simulink model "MCVT_Integration"  (R2020a)
settings: Solver=ode8  StartTime=0.0  StopTime=T_end  FixedStep=dt  RelTol=1e-3  EnableMultiTasking=on
values from neighbouring files (symbols this model uses):
  assumed_power_voltage = 1.25e2    (= 125)    [MCVT_Input_File.m]    (also in MCVT_Run_QuickCheck.m)
  battery_power_toggle = 1    [MCVT_Input_File.m]    (also in MCVT_Run_QuickCheck.m)
  battery_trickle_charge_param = 0.1    [MCVT_Input_File.m]    (also in MCVT_Run_QuickCheck.m)
  battery_trickle_leakage_param = 0.01    [MCVT_Input_File.m]    (also in MCVT_Run_QuickCheck.m)
  damage_case_id = 3    [MCVT_Input_File.m]    (also in MCVT_Run_QuickCheck.m = 1)
  dt = 1/fs    (= 0.001)    [MCVT_Run_QuickCheck.m]    (also in MCVT_Sim_Set.m)
  dt_2 = 1/fs_2    (= 0.001)    [MCVT_Run_QuickCheck.m]    (also in MCVT_Sim_Set.m)
  dt_3 = 1/fs_3    (= 0.001)    [MCVT_Run_QuickCheck.m]    (also in MCVT_Sim_Set.m)
  dt_5 = 1/fs_5    (= 0.005)    [MCVT_Run_QuickCheck.m]    (also in MCVT_Sim_Set.m)
  dt_8 = 1/fs_8    (= 0.05)    [MCVT_Run_QuickCheck.m]    (also in MCVT_Sim_Set.m)
  dt_9 = 1/fs_9    (= 0.001)    [MCVT_Run_QuickCheck.m]    (also in MCVT_Sim_Set.m)
  dust_met_duration = 3    [MCVT_Input_File.m]    (also in MCVT_Run_QuickCheck.m = 2)
  ECLSS_indoor_set_temperature = 298.15    [MCVT_Input_File.m]    (also in MCVT_Run_QuickCheck.m)
  energy_store_max_o = 150    [MCVT_Input_File.m]    (also in MCVT_Run_QuickCheck.m)
  energy_store_o = 120    [MCVT_Input_File.m]    (also in MCVT_Run_QuickCheck.m)
  fs = 1000    [MCVT_Run_QuickCheck.m]    (also in MCVT_Sim_Set.m)
  gain_charge_param = 1    [MCVT_Input_File.m]    (also in MCVT_Run_QuickCheck.m)
  ground_temperature = 373    [MCVT_Habitat_Design_Input_File.m]    (also in MCVT_Run_QuickCheck.m)
  I_max_charge = 800    [MCVT_Input_File.m]    (also in MCVT_Run_QuickCheck.m)
  I_max_discharge = 800    [MCVT_Input_File.m]    (also in MCVT_Run_QuickCheck.m)
  initial_pressure = 1e5    (= 100000)    [MCVT_Input_File.m]    (also in MCVT_Run_QuickCheck.m)
  initial_temperature = 295    [MCVT_Input_File.m]    (also in MCVT_Run_QuickCheck.m)
  interior_leak_rate = 0.00415    [MCVT_Input_File.m]    (also in MCVT_Run_QuickCheck.m)
  launch_dust_accum_start_time = 10    [MCVT_Input_File.m]    (also in MCVT_Run_QuickCheck.m = 2)
  launch_dust_accum_stop_time = 12    [MCVT_Input_File.m]    (also in MCVT_Run_QuickCheck.m = 3)
  meteorite_impact_time = 2    [MCVT_Input_File.m]    (also in MCVT_Run_QuickCheck.m = 1)
  nuclear_power_toggle = 1    [MCVT_Input_File.m]    (also in MCVT_Run_QuickCheck.m)
  print_interval = 30    [MCVT_Input_File.m]
  SOC_lower_thres = 0.5    [MCVT_Input_File.m]    (also in MCVT_Run_QuickCheck.m)
  SOC_upper_thres = 0.9    [MCVT_Input_File.m]    (also in MCVT_Run_QuickCheck.m)
  solar_power_toggle = 1    [MCVT_Input_File.m]    (also in MCVT_Run_QuickCheck.m)
  space_temperature = 4    [MCVT_Habitat_Design_Input_File.m]    (also in MCVT_Run_QuickCheck.m)
  Start_angle = 90    [MCVT_Input_File.m]    (also in MCVT_Run_QuickCheck.m)
undefined symbols (not defined in the file or in its folder): A_flow_restrictor, ACTIVITY_LOOKUP_TABLE, air, Aref, Bmap, Bmap2, Bmap3, comms, DiscIntNeverNeededParam, DmgSPL, dust_parameters, ECLSS_dust_damage_repair_time, ECLSS_heater_capacity, ECLSS_IPS_restrictor, ECLSS_IPS_setpointPressure, ECLSS_paint_damage_repair_time, ECLSS_radiatorLoop_discreteNumber, ECLSS_Vst, emptyActivity, expected_heat_add, FI, habitat, HIEM2SMM, HIEM2STM, HIEMDamageElements, impact_numbering, initial_SPLT_damage_index, input_air, input_Cond, input_CP, input_discretization, input_EV, input_Evap, input_radiatorLoop, K_ECLSS_dust_single, K_ECLSS_paint_single, K_Structure_damage_single, Kref, kW_to_W, meteoroidDustDep, mFRF, ndelays, nominalDustDep, noverlap, nuclear_toggle_gain, output_limits, panel_paint0, Ps0, reference_temperature, RepairTime_ECLSS_factor, Sensor_Location_SMM, SMM_T0, SMM_T1, SMM_T2, SMM_T3, SMM_T4, SMM_T4_0, SMM_T4_1, SMM_T4_2, solar_FDD_K ... (+73)
libraries: neural x350, simulink x39, xpcutilitieslib x7, xpcrtudplib x7, dspstat3 x1
(part 20 of 43)

== system MCVT_Integration/Sys2 - Structural System/Sys2_Structural_System/Fault Detection and Diagnosis (FDD) 2.3/Pattern Recognition Neural Network/Pattern Recognition Neural Network with 10% Noise/Layer 2/Delays 1 ==
  "p{2}" : Inport  PortDimensions=20
      mask Display: e = 0.06;c1 = [120 230 180]/255;c2 = ([120 230 180]+0)/(256+128);c3 = ([120 230 180]+128)/(256+128);patch([0 1 1 0],[0 0 1 1],c1);patch([0 1 1 1-e 1-e 0+e],[0 0 1 1-e 0+e 0+e],c2);patch([0 0 1 1-e 0+e 0+e],[0 1 1 1-e 1-e 0+e],c3);plot([0 1 1 0 0],[0 0 1 1 0]);
  mux : Mux  Inputs=1
      mask Display: e = 0.06;c1 = [120 150 230]/255;c2 = ([120 150 230]+0)/383;c3 = ([120 150 230]+128)/383;patch([0 1 1 0],[0 0 1 1],c1);patch([0 1 1 1-e 1-e 0+e],[0 0 1 1-e 0+e 0+e],c2);patch([0 0 1 1-e 0+e 0+e],[0 1 1 1-e 1-e 0+e],c3);plot([0 1 1 0 0],[0 0 1 1 0]);disp('Mux')
  "pd{2,1}" : Outport
      mask Display: e = 0.06;c1 = [120 230 180]/255;c2 = ([120 230 180]+0)/(256+128);c3 = ([120 230 180]+128)/(256+128);patch([0 1 1 0],[0 0 1 1],c1);patch([0 1 1 1-e 1-e 0+e],[0 0 1 1-e 0+e 0+e],c2);patch([0 0 1 1-e 0+e 0+e],[0 1 1 1-e 1-e 0+e],c3);plot([0 1 1 0 0],[0 0 1 1 0]);
  wires:
  "p{2}"/1 -> mux/1
  mux/1 -> "pd{2,1}"/1

== system MCVT_Integration/Sys2 - Structural System/Sys2_Structural_System/Fault Detection and Diagnosis (FDD) 2.3/Pattern Recognition Neural Network/Pattern Recognition Neural Network with 10% Noise/Layer 2/LW{2,1} ==
  "ad{2,1}" : Inport  PortDimensions=20
      mask Display: e = 0.06;c1 = [120 230 180]/255;c2 = ([120 230 180]+0)/(256+128);c3 = ([120 230 180]+128)/(256+128);patch([0 1 1 0],[0 0 1 1],c1);patch([0 1 1 1-e 1-e 0+e],[0 0 1 1-e 0+e 0+e],c2);patch([0 0 1 1-e 0+e 0+e],[0 1 1 1-e 1-e 0+e],c3);plot([0 1 1 0 0],[0 0 1 1 0]);
  "IW{2,1}(1,:)'" : Constant  Value=[-0.574271628074736195657123971614055335521697998046875;0.50810117268876220375517505090101622045040130615234375;-0.9283778525542050186203368866699747741222381591796875;-0.032683118602653167539262568652702611871063709259033203125;-0.0145954644987950805934406872665931587107479572296142578125;0.443944394435375422336420569990877993404865264892578125;0.4214764366206338319642554779420606791973114013671875;-0.386523310467667136069991329350159503519535064697265625;-0.25850103440934268927975381302530877292156219482421875;-0.91087816091310769284206116935820318758487701416015625;-0.1963259746056559562621401937576592899858951568603515625;-0.1813018586134855503200213888703729026019573211669921875;-0.49254604106108257344232015384477563202381134033203125;-0.394272569002943440441555367215187288820743560791015625;-0.062085423077329747465480380697044893167912960052490234375;-0.041503725787217782272620070216362364590167999267578125;0.1806376114545178435122352311736904084682464599609375;0.164222888558606339159950948669575154781341552734375;-0.5222337211202112694508059576037339866161346435546875;-0.441330444361871021552445881752646528184413909912109375]
      mask Display: e = 0.06;c1 = [120 150 230]/255;c2 = ([120 150 230]+0)/383;c3 = ([120 150 230]+128)/383;patch([0 1 1 0],[0 0 1 1],c1);patch([0 1 1 1-e 1-e 0+e],[0 0 1 1-e 0+e 0+e],c2);patch([0 0 1 1-e 0+e 0+e],[0 1 1 1-e 1-e 0+e],c3);plot([0 1 1 0 0],[0 0 1 1 0]);disp('weights');
  "IW{2,1}(10,:)'" : Constant  Value=[0.56889257212312560607614386753994040191173553466796875;0.1885837971524775558140873954471317119896411895751953125;0.6485295796017667147026486418326385319232940673828125;-0.451249687405113453220195651738322339951992034912109375;-0.3971195859441050135529849285376258194446563720703125;0.427965433320891597279711504597798921167850494384765625;-0.656993381392033892751669554854743182659149169921875;-0.0936009421839196498194013429383630864322185516357421875;-0.234845048198842609732395203536725603044033050537109375;-0.84261949124741220007450692719430662691593170166015625;0.3092932033834203675581875359057448804378509521484375;-0.69446240960240535056158250881708227097988128662109375;0.133242084727271781208202128254924900829792022705078125;0.49326867993430412173694321609218604862689971923828125;-0.06733328074539456087332922606947249732911586761474609375;-0.278535206843569416168548968926188535988330841064453125;0.277173212586397099688184653132339008152484893798828125;0.168172076864115560557166872968082316219806671142578125;-0.338431949921502994715893919419613666832447052001953125;0.020035112474400061655099847257588407956063747406005859375]
      mask Display: e = 0.06;c1 = [120 150 230]/255;c2 = ([120 150 230]+0)/383;c3 = ([120 150 230]+128)/383;patch([0 1 1 0],[0 0 1 1],c1);patch([0 1 1 1-e 1-e 0+e],[0 0 1 1-e 0+e 0+e],c2);patch([0 0 1 1-e 0+e 0+e],[0 1 1 1-e 1-e 0+e],c3);plot([0 1 1 0 0],[0 0 1 1 0]);disp('weights');
  "IW{2,1}(11,:)'" : Constant  Value=[-0.61220927358305521348569300243980251252651214599609375;-0.35057890410002789938204159625456668436527252197265625;-0.523626035897452712930544294067658483982086181640625;0.436194545470680983267897090627229772508144378662109375;0.1213313887947793101584892383471014909446239471435546875;-0.0121676389988455875557082919158347067423164844512939453125;-0.0633751033433716803866531108724302612245082855224609375;-0.54877334524708165641726509420550428330898284912109375;0.1756665111292425029976271844134316779673099517822265625;0.52479855734203051387254390647285617887973785400390625;0.31253261939911680666881466095219366252422332763671875;-0.061689810511481708854564232069606077857315540313720703125;-0.36854617261526312343988820430240593850612640380859375;0.400723045351511986122972075463621877133846282958984375;-0.1532168475451168021539416486120899207890033721923828125;-0.64645781400316526532634497925755567848682403564453125;0.5697695890396656803744690478197298943996429443359375;0.1448942726284534188163632961732218973338603973388671875;-0.438188292108895705023741129480185918509960174560546875;-0.2074698900457048467149689940924872644245624542236328125]
      mask Display: e = 0.06;c1 = [120 150 230]/255;c2 = ([120 150 230]+0)/383;c3 = ([120 150 230]+128)/383;patch([0 1 1 0],[0 0 1 1],c1);patch([0 1 1 1-e 1-e 0+e],[0 0 1 1-e 0+e 0+e],c2);patch([0 0 1 1-e 0+e 0+e],[0 1 1 1-e 1-e 0+e],c3);plot([0 1 1 0 0],[0 0 1 1 0]);disp('weights');
  "IW{2,1}(12,:)'" : Constant  Value=[-0.05684625507318873116613389129270217381417751312255859375;0.7124306870197238961139873936190269887447357177734375;-0.0255329045034950428971765035157659440301358699798583984375;0.2385497059408359932231036282246350310742855072021484375;0.97337720939202998504669039903092198073863983154296875;0.3646568944777561771530827172682620584964752197265625;0.54797761780701914613445069335284642875194549560546875;0.37164823961946014119206438408582471311092376708984375;-0.08512149605261863649641185247674002312123775482177734375;-0.09309100636109647608495976101039559580385684967041015625;0.10445071447821853427573302042219438590109348297119140625;0.450251121221431793717471236959681846201419830322265625;-0.315036492181973659665317200051504187285900115966796875;0.2069138675097972335237273000529967248439788818359375;-0.07269256325197571977358990125139825977385044097900390625;-0.358315402073627942147737712730304338037967681884765625;0.00748683608178967417712268428431343636475503444671630859375;0.63657745039067881176464425152516923844814300537109375;-0.0188097270602836132147306358319838182069361209869384765625;-0.435424821932042849237376458404469303786754608154296875]
      mask Display: e = 0.06;c1 = [120 150 230]/255;c2 = ([120 150 230]+0)/383;c3 = ([120 150 230]+128)/383;patch([0 1 1 0],[0 0 1 1],c1);patch([0 1 1 1-e 1-e 0+e],[0 0 1 1-e 0+e 0+e],c2);patch([0 0 1 1-e 0+e 0+e],[0 1 1 1-e 1-e 0+e],c3);plot([0 1 1 0 0],[0 0 1 1 0]);disp('weights');
  "IW{2,1}(13,:)'" : Constant  Value=[-0.005992500139532979351597585804256596020422875881195068359375;-0.4560900715520570525285393159720115363597869873046875;-0.306276633508657025739552182130864821374416351318359375;0.5216871924691381945393686692113988101482391357421875;-0.316654318650212707186852867380366660654544830322265625;0.1121328110068039174418430548030300997197628021240234375;0.093640236792591424741516448193578980863094329833984375;0.17502083406653123898166768412920646369457244873046875;-0.60837609782212742626228418885148130357265472412109375;0.357162613418083640937084055622108280658721923828125;0.64597875254259007338220044402987696230411529541015625;-0.412303583049930477688604923969251103699207305908203125;-0.29760774376247056682842639929731376469135284423828125;-0.306518613632401681901029633081634528934955596923828125;0.26479518516167266373173561078147031366825103759765625;0.1475845467874192518120679551429930143058300018310546875;-0.273480753422654820905535189012880437076091766357421875;0.634244403850074522921431707800365984439849853515625;-0.2405905212879165533390590780982165597379207611083984375;0.042143875875917773121681619841183419339358806610107421875]
      mask Display: e = 0.06;c1 = [120 150 230]/255;c2 = ([120 150 230]+0)/383;c3 = ([120 150 230]+128)/383;patch([0 1 1 0],[0 0 1 1],c1);patch([0 1 1 1-e 1-e 0+e],[0 0 1 1-e 0+e 0+e],c2);patch([0 0 1 1-e 0+e 0+e],[0 1 1 1-e 1-e 0+e],c3);plot([0 1 1 0 0],[0 0 1 1 0]);disp('weights');
  "IW{2,1}(14,:)'" : Constant  Value=[0.83050427742647514595120128433336503803730010986328125;0.380791907424027631368090851537999697029590606689453125;0.64991803081990628943032106690225191414356231689453125;-0.08751040212893225478385517135393456555902957916259765625;-0.304332767679233062718680002944893203675746917724609375;-0.54612464997613496819894862710498273372650146484375;-0.35114844609709716660717049308004789054393768310546875;-0.348036230611717234406654597478336654603481292724609375;-0.53144460799473625112199215436703525483608245849609375;-0.0777327876198296296994527665447094477713108062744140625;-0.345073957804460318410377794862142764031887054443359375;-0.205600532452644924052975738959503360092639923095703125;-0.049181910233272840837770445432397536933422088623046875;0.250885063190241563990667827965808100998401641845703125;0.43391138223406156004813283288967795670032501220703125;-0.1461886322383406378033754435818991623818874359130859375;0.6178452278896970906174601623206399381160736083984375;0.266994784317448774491055019097984768450260162353515625;-0.49335961290386498578897089828387834131717681884765625;0.022843510039939569911116024059083429165184497833251953125]
      mask Display: e = 0.06;c1 = [120 150 230]/255;c2 = ([120 150 230]+0)/383;c3 = ([120 150 230]+128)/383;patch([0 1 1 0],[0 0 1 1],c1);patch([0 1 1 1-e 1-e 0+e],[0 0 1 1-e 0+e 0+e],c2);patch([0 0 1 1-e 0+e 0+e],[0 1 1 1-e 1-e 0+e],c3);plot([0 1 1 0 0],[0 0 1 1 0]);disp('weights');
  "IW{2,1}(15,:)'" : Constant  Value=[0.394338359224950363302042433133465237915515899658203125;-0.324812785108549439971881156452582217752933502197265625;0.0334474272752659229634986104429117403924465179443359375;-0.113914753942840007994163897819817066192626953125;0.5702661865720821676717378068133257329463958740234375;-0.56902393479980106771876080529182218015193939208984375;-1.080364855197034845701864469447173178195953369140625;-0.43915404493278786812737735090195201337337493896484375;0.02888946354385936443609494972406537272036075592041015625;0.360330786452452256352074755341163836419582366943359375;-0.472775036000186776874443239648826420307159423828125;0.5191143553324497617040833574719727039337158203125;0.05346616409203776287828446811545290984213352203369140625;-0.108658401740322874129418551092385314404964447021484375;0.67532661092200696462128917119116522371768951416015625;-0.5013587075398808767801028807298280298709869384765625;0.393844819705647442020790549577213823795318603515625;0.412756827570472217825425786941195838153362274169921875;-0.55847529512976767929188781636185012757778167724609375;-0.205862070370640803940176510877790860831737518310546875]
      mask Display: e = 0.06;c1 = [120 150 230]/255;c2 = ([120 150 230]+0)/383;c3 = ([120 150 230]+128)/383;patch([0 1 1 0],[0 0 1 1],c1);patch([0 1 1 1-e 1-e 0+e],[0 0 1 1-e 0+e 0+e],c2);patch([0 0 1 1-e 0+e 0+e],[0 1 1 1-e 1-e 0+e],c3);plot([0 1 1 0 0],[0 0 1 1 0]);disp('weights');
  "IW{2,1}(16,:)'" : Constant  Value=[0.27635654239410623045358761373790912330150604248046875;-0.04429311840556114654443575773257180117070674896240234375;0.5431988410510484310833589916001074016094207763671875;-0.57140306934570206376378109780489467084407806396484375;0.28768730719099533388316558557562530040740966796875;0.5939455878345356598657645008643157780170440673828125;0.07670371326740492434748119876530836336314678192138671875;0.592025013385914622432437681709416210651397705078125;-0.12346456437285503737744107866092235781252384185791015625;0.40383872281908173018649677032954059541225433349609375;0.1638374993707522320196545706494362093508243560791015625;-0.233077013410165623241709909052588045597076416015625;-0.382987569183739273714905948509112931787967681884765625;0.237088361565319261092810165791888721287250518798828125;-0.032614993468910190299592244400628260336816310882568359375;0.18972721693147354127262360634631477296352386474609375;0.477835629992515276143194569158367812633514404296875;-0.444967139434641223427746581364772282540798187255859375;0.36956516035198128111716187049751169979572296142578125;0.260159387876649272897111586644314229488372802734375]
      mask Display: e = 0.06;c1 = [120 150 230]/255;c2 = ([120 150 230]+0)/383;c3 = ([120 150 230]+128)/383;patch([0 1 1 0],[0 0 1 1],c1);patch([0 1 1 1-e 1-e 0+e],[0 0 1 1-e 0+e 0+e],c2);patch([0 0 1 1-e 0+e 0+e],[0 1 1 1-e 1-e 0+e],c3);plot([0 1 1 0 0],[0 0 1 1 0]);disp('weights');
  "IW{2,1}(17,:)'" : Constant  Value=[-0.10300827207069433721908779943987610749900341033935546875;-0.062365882473237134464572051228969939984381198883056640625;-0.0506656936086894160720106583539745770394802093505859375;0.400652635851392491250777538880356587469577789306640625;-0.64362923376520597695815695260534994304180145263671875;-0.10550968910703635572634340178410639055073261260986328125;-0.436641866892334473693182417264324612915515899658203125;0.2877789503365608680951481801457703113555908203125;-0.0856422481667453439069248588566551916301250457763671875;-0.0352973610691887651480413978788419626653194427490234375;0.251271296193900373072693810172495432198047637939453125;0.158396296935443603448590010884799994528293609619140625;-0.02336399796777975657047221602624631486833095550537109375;0.2405152682774551464728318705965648405253887176513671875;-0.422851332433710036529106446323567070066928863525390625;0.0545271257407160536700985176139511168003082275390625;0.63574596562661944698646721008117310702800750732421875;0.71382341994933418138913339134887792170047760009765625;-0.5918051425522603548046163268736563622951507568359375;0.18430848892072082545467992531484924256801605224609375]
      mask Display: e = 0.06;c1 = [120 150 230]/255;c2 = ([120 150 230]+0)/383;c3 = ([120 150 230]+128)/383;patch([0 1 1 0],[0 0 1 1],c1);patch([0 1 1 1-e 1-e 0+e],[0 0 1 1-e 0+e 0+e],c2);patch([0 0 1 1-e 0+e 0+e],[0 1 1 1-e 1-e 0+e],c3);plot([0 1 1 0 0],[0 0 1 1 0]);disp('weights');
  "IW{2,1}(18,:)'" : Constant  Value=[-0.25446115074283692791823341394774615764617919921875;-0.476422817454355607136307071414194069802761077880859375;-0.48243039010070898342519285506568849086761474609375;0.12240083511392434167941445366523112170398235321044921875;0.370582478256486547163461864329292438924312591552734375;-0.565112313381039399473593221046030521392822265625;0.1811578060472032458694258139075827784836292266845703125;-0.385885379099205449993092997829080559313297271728515625;0.1234837116886016994410368852186365984380245208740234375;0.271571689696240070599486671198974363505840301513671875;0.26568034152349895293809822760522365570068359375;-0.36135745586302314702464855145080946385860443115234375;-0.2479618589940788542236305147525854408740997314453125;-0.465725675460194776800193494636914692819118499755859375;-0.029786737886606184932958285571658052504062652587890625;0.10761968787180224704780329147979500703513622283935546875;-0.39997554837126070292896429236861877143383026123046875;0.47018260234583897361204662956879474222660064697265625;0.474125103036238948472913534715189598500728607177734375;0.5711459194855550425273804648895747959613800048828125]
      mask Display: e = 0.06;c1 = [120 150 230]/255;c2 = ([120 150 230]+0)/383;c3 = ([120 150 230]+128)/383;patch([0 1 1 0],[0 0 1 1],c1);patch([0 1 1 1-e 1-e 0+e],[0 0 1 1-e 0+e 0+e],c2);patch([0 0 1 1-e 0+e 0+e],[0 1 1 1-e 1-e 0+e],c3);plot([0 1 1 0 0],[0 0 1 1 0]);disp('weights');
  "IW{2,1}(19,:)'" : Constant  Value=[-0.63386779810769622311994453411898575723171234130859375;-0.50314690781331872226900259192916564643383026123046875;0.268331211259087709652959574668784625828266143798828125;-0.1955754337239898887901290436275303363800048828125;-0.35669681164923139338185364977107383310794830322265625;0.502236421766609542061132742674089968204498291015625;-0.11159206649818120826722633864847011864185333251953125;0.436217158032130292166783647189731709659099578857421875;-0.27584823963679705460805280381464399397373199462890625;0.418362204883860833160014180975849740207195281982421875;-0.1899008077758812529367560273385606706142425537109375;0.57483413850714948356568356757634319365024566650390625;-0.446857134984141424194348246601293794810771942138671875;0.396945475581674422915767763697658665478229522705078125;-1.0613420562751685327640416289796121418476104736328125;-0.285221282032167178233095228279125876724720001220703125;0.45693712919073814493486906940233893692493438720703125;0.07744397689539132823011158279769006185233592987060546875;0.354164362297970491955112493087654002010822296142578125;-0.0162778031372035510149220982611950603313744068145751953125]
      mask Display: e = 0.06;c1 = [120 150 230]/255;c2 = ([120 150 230]+0)/383;c3 = ([120 150 230]+128)/383;patch([0 1 1 0],[0 0 1 1],c1);patch([0 1 1 1-e 1-e 0+e],[0 0 1 1-e 0+e 0+e],c2);patch([0 0 1 1-e 0+e 0+e],[0 1 1 1-e 1-e 0+e],c3);plot([0 1 1 0 0],[0 0 1 1 0]);disp('weights');
  "IW{2,1}(2,:)'" : Constant  Value=[0.170738862388308543227566360656055621802806854248046875;-0.268815089772659943090360457063070498406887054443359375;-0.38720939538457821438299788496806286275386810302734375;0.01483966791631497784964555108899730839766561985015869140625;-0.486697009966023108606947289445088244974613189697265625;0.309172138529930418116009605000726878643035888671875;0.06373514748131080265292069952920428477227687835693359375;-0.60590099369459993194908520308672450482845306396484375;0.35640677444520363348345881604473106563091278076171875;-0.5909803027849054313236365487682633101940155029296875;-0.1285903836946735712132294793264009058475494384765625;0.4466134666669081543233232878264971077442169189453125;-0.1984531065465708066231087514097453095018863677978515625;0.325192889511320359918755684702773578464984893798828125;0.47371923459628739738747071896796114742755889892578125;0.593039767991454969120468376786448061466217041015625;0.345064373904851395824522342081763781607151031494140625;0.1563268051332172559053645954918465577065944671630859375;0.2085629962423511674085574441050994209945201873779296875;0.2477676431329221828914199932114570401608943939208984375]
      mask Display: e = 0.06;c1 = [120 150 230]/255;c2 = ([120 150 230]+0)/383;c3 = ([120 150 230]+128)/383;patch([0 1 1 0],[0 0 1 1],c1);patch([0 1 1 1-e 1-e 0+e],[0 0 1 1-e 0+e 0+e],c2);patch([0 0 1 1-e 0+e 0+e],[0 1 1 1-e 1-e 0+e],c3);plot([0 1 1 0 0],[0 0 1 1 0]);disp('weights');
  "IW{2,1}(20,:)'" : Constant  Value=[0.034625411356367034876324595416008378379046916961669921875;0.1874616562450983348053767940655234269797801971435546875;0.04914463536819181632697706163526163436472415924072265625;-0.59175777311133870295378756054560653865337371826171875;-0.90960536825278170969255597810843028128147125244140625;0.32990973138561152655512387354974634945392608642578125;0.06897862063135924703960455417472985573112964630126953125;0.3352281455569865453725242332438938319683074951171875;0.56131365538922206592786778855952434241771697998046875;0.5368981405184618527215434369281865656375885009765625;-0.332503821804824628571850553271360695362091064453125;0.277925405078486231769119285672786645591259002685546875;-0.3078968918083386796524791861884295940399169921875;0.423244800153884437321494260686449706554412841796875;-0.492707237515911000702573119269800372421741485595703125;-0.124415208625734130531981236345018260180950164794921875;0.401670717019710299400259145841118879616260528564453125;0.254209817383537262092119135559187270700931549072265625;-0.335112590406773491746861282081226818263530731201171875;0.69289987289486221211376459905295632779598236083984375]
      mask Display: e = 0.06;c1 = [120 150 230]/255;c2 = ([120 150 230]+0)/383;c3 = ([120 150 230]+128)/383;patch([0 1 1 0],[0 0 1 1],c1);patch([0 1 1 1-e 1-e 0+e],[0 0 1 1-e 0+e 0+e],c2);patch([0 0 1 1-e 0+e 0+e],[0 1 1 1-e 1-e 0+e],c3);plot([0 1 1 0 0],[0 0 1 1 0]);disp('weights');
  "IW{2,1}(3,:)'" : Constant  Value=[-0.68384728468662192568672253401018679141998291015625;-0.18667975716109364014982929802499711513519287109375;-0.5796974119018820648108203386073000729084014892578125;0.444457322623959816265681865843362174928188323974609375;0.3358991937804882699225572650902904570102691650390625;0.62509683106080216052902187584550119936466217041015625;-0.2121453725660192024538019950341549701988697052001953125;0.1528299410147090087175314465639530681073665618896484375;-0.26522546290072701591356008066213689744472503662109375;-0.833329512393990778917896022903732955455780029296875;0.0245823899162304800036782381766897742636501789093017578125;0.3242450158499738943618240227806381881237030029296875;0.207550979496584797612257489163312129676342010498046875;-0.5781973676120577554371493533835746347904205322265625;0.84734773074372016221644798861234448850154876708984375;0.08584960360003891788327479162035160697996616363525390625;-0.5281438899797261665725045531871728599071502685546875;-0.377490389258728520349706059278105385601520538330078125;-0.255276769465087205457365371330524794757366180419921875;0.397482914908264428621720298906438983976840972900390625]
      mask Display: e = 0.06;c1 = [120 150 230]/255;c2 = ([120 150 230]+0)/383;c3 = ([120 150 230]+128)/383;patch([0 1 1 0],[0 0 1 1],c1);patch([0 1 1 1-e 1-e 0+e],[0 0 1 1-e 0+e 0+e],c2);patch([0 0 1 1-e 0+e 0+e],[0 1 1 1-e 1-e 0+e],c3);plot([0 1 1 0 0],[0 0 1 1 0]);disp('weights');
  "IW{2,1}(4,:)'" : Constant  Value=[-0.469126351790188966806027792699751444160938262939453125;0.65369714251824551087821646433440037071704864501953125;-0.62799203253491719944889837279333733022212982177734375;-0.2351882475270932193467388060525991022586822509765625;-0.11164975269919773237514704078421345911920070648193359375;-0.493471669872333873474445908868801780045032501220703125;0.9180235431853904781718256344902329146862030029296875;0.07102998928975334802515817500534467399120330810546875;0.316111646796484568877616538884467445313930511474609375;0.47279397677343915074033020573551766574382781982421875;0.39848637281463583637020064998068846762180328369140625;-0.85560991819153253690188876134925521910190582275390625;-0.415615780819191649175792235837434418499469757080078125;0.32152173865090272197875265192124061286449432373046875;0.58442540995306024864675009666825644671916961669921875;-0.1628689347883164606489714287818060256540775299072265625;-0.2123151870600946955480736733079538680613040924072265625;0.36373178934589844857327989302575588226318359375;-0.096673513630073759639316222092020325362682342529296875;-0.423399450849545611053059701589518226683139801025390625]
      mask Display: e = 0.06;c1 = [120 150 230]/255;c2 = ([120 150 230]+0)/383;c3 = ([120 150 230]+128)/383;patch([0 1 1 0],[0 0 1 1],c1);patch([0 1 1 1-e 1-e 0+e],[0 0 1 1-e 0+e 0+e],c2);patch([0 0 1 1-e 0+e 0+e],[0 1 1 1-e 1-e 0+e],c3);plot([0 1 1 0 0],[0 0 1 1 0]);disp('weights');
  "IW{2,1}(5,:)'" : Constant  Value=[0.467549098114494021682929769667680375277996063232421875;0.827698304288310371390480213449336588382720947265625;0.702652589865448984340901006362400949001312255859375;-0.51130807898153129986695830666576512157917022705078125;0.6848509081607254689316732765291817486286163330078125;0.3376259025690153681154015430365689098834991455078125;0.519716199692204394722239157999865710735321044921875;0.28040092188565879371964228994329459965229034423828125;-0.689298003344263054970042503555305302143096923828125;-0.0105352229071583626540675737714991555549204349517822265625;0.11065686698639161378299178295492311008274555206298828125;0.483571718741354406034815838211216032505035400390625;0.015877326853821822905121763369606924243271350860595703125;-0.341319976275820169409058735254802741110324859619140625;0.58630660169135551118557714289636351168155670166015625;-0.5351595353677094379918344202451407909393310546875;-0.09620277639251866019787229333815048448741436004638671875;-0.55510963521854417290768424209090881049633026123046875;-0.37198801247729951224840760914958082139492034912109375;-0.327000641897117250156412637807079590857028961181640625]
      mask Display: e = 0.06;c1 = [120 150 230]/255;c2 = ([120 150 230]+0)/383;c3 = ([120 150 230]+128)/383;patch([0 1 1 0],[0 0 1 1],c1);patch([0 1 1 1-e 1-e 0+e],[0 0 1 1-e 0+e 0+e],c2);patch([0 0 1 1-e 0+e 0+e],[0 1 1 1-e 1-e 0+e],c3);plot([0 1 1 0 0],[0 0 1 1 0]);disp('weights');
  "IW{2,1}(6,:)'" : Constant  Value=[-0.3453678687030630189980229260982014238834381103515625;0.48523303772118564491933057070127688348293304443359375;0.4640167040202658466085949839907698333263397216796875;0.144233208507599408587651623747660778462886810302734375;0.493274737844617783277811895459308288991451263427734375;-0.416272467860007655549026139851775951683521270751953125;-0.2153817688011791797730865027915569953620433807373046875;-0.2132520628956689756616782460696413181722164154052734375;-0.1452665872457030438180680675941403023898601531982421875;0.72743294568573768632546716617071069777011871337890625;-0.0156095830614016162218860017674160189926624298095703125;-0.55213857512219599588121354827308095991611480712890625;-0.478229271237840591890488894932786934077739715576171875;-0.07855489886809836896919279070061747916042804718017578125;0.71482623751985574500622533378191292285919189453125;-0.07307442229493234242543309164830134250223636627197265625;0.021302672650072733417925263665892998687922954559326171875;0.1991062808234271008789306733888224698603153228759765625;-0.016061880453676256885753304004538222216069698333740234375;0.49043374611209333835404322599060833454132080078125]
      mask Display: e = 0.06;c1 = [120 150 230]/255;c2 = ([120 150 230]+0)/383;c3 = ([120 150 230]+128)/383;patch([0 1 1 0],[0 0 1 1],c1);patch([0 1 1 1-e 1-e 0+e],[0 0 1 1-e 0+e 0+e],c2);patch([0 0 1 1-e 0+e 0+e],[0 1 1 1-e 1-e 0+e],c3);plot([0 1 1 0 0],[0 0 1 1 0]);disp('weights');
  "IW{2,1}(7,:)'" : Constant  Value=[0.72351439888291457780411519706831313669681549072265625;-0.1321783567282871219017437169895856641232967376708984375;-0.28471936669232877914481605330365709960460662841796875;0.061886306878214621118861060722338152118027210235595703125;0.044082279699798589123194147987305768765509128570556640625;-1.0058490357309965990140199210145510733127593994140625;0.007906537149789762131124604138676659204065799713134765625;-0.14891864701538304327499417922808788716793060302734375;-0.89324719250629869282676054353942163288593292236328125;-0.43011400335492255830871499711065553128719329833984375;0.2189712711045234960760552667125011794269084930419921875;0.299675210484005527522555212271981872618198394775390625;0.166576896869622570473978839800111018121242523193359375;0.458167405913204872636157460874528624117374420166015625;-0.90127909080610957293089313679956831037998199462890625;0.66265998166458228713082689864677377045154571533203125;0.179000288337995250298462224236573092639446258544921875;0.4549568523737634873072011032490991055965423583984375;0.3212188486964453471017577612656168639659881591796875;0.0669570174398022022188570190337486565113067626953125]
      mask Display: e = 0.06;c1 = [120 150 230]/255;c2 = ([120 150 230]+0)/383;c3 = ([120 150 230]+128)/383;patch([0 1 1 0],[0 0 1 1],c1);patch([0 1 1 1-e 1-e 0+e],[0 0 1 1-e 0+e 0+e],c2);patch([0 0 1 1-e 0+e 0+e],[0 1 1 1-e 1-e 0+e],c3);plot([0 1 1 0 0],[0 0 1 1 0]);disp('weights');
  "IW{2,1}(8,:)'" : Constant  Value=[-0.24003561318120636425277325542992912232875823974609375;0.360479003035218903416847524567856453359127044677734375;-0.2152665740272724781423363538124249316751956939697265625;0.52459394622592780965675274273962713778018951416015625;-0.42728186204076068488433293168782256543636322021484375;0.282699647456826352875935981501243077218532562255859375;0.48875871512639934568511534962453879415988922119140625;-0.334216509402603778600138184629031457006931304931640625;0.62795665865529526339372523580095730721950531005859375;0.258406605325758487179399480737629346549510955810546875;0.0246770370671213733204663043352411477826535701751708984375;0.05090768838384629457749497305485419929027557373046875;-0.235335248267196817106849948686431162059307098388671875;-0.3326875554275947610705088663962669670581817626953125;0.64438444567001418317175875927205197513103485107421875;0.34644257998336336346056896218215115368366241455078125;-0.4826091697402272728822936187498271465301513671875;0.2327352134993600218937359613846638239920139312744140625;0.11929884331874819614061067341026500798761844635009765625;-0.53619405868680358739908342613489367067813873291015625]
      mask Display: e = 0.06;c1 = [120 150 230]/255;c2 = ([120 150 230]+0)/383;c3 = ([120 150 230]+128)/383;patch([0 1 1 0],[0 0 1 1],c1);patch([0 1 1 1-e 1-e 0+e],[0 0 1 1-e 0+e 0+e],c2);patch([0 0 1 1-e 0+e 0+e],[0 1 1 1-e 1-e 0+e],c3);plot([0 1 1 0 0],[0 0 1 1 0]);disp('weights');
  "IW{2,1}(9,:)'" : Constant  Value=[-0.6912593856225310862129163069766946136951446533203125;0.380992328274060820803015303681604564189910888671875;-0.08471902480088629172616521145755541510879993438720703125;0.36149205929553207550242177603649906814098358154296875;-0.35013229998088568617475857536192052066326141357421875;-0.07974761403618869926024359529037610627710819244384765625;-0.80823258236509609719888658219133503735065460205078125;0.254955742284075970172096958776819519698619842529296875;0.18794697818350247775498473856714554131031036376953125;0.2196563917977779889501022125841700471937656402587890625;0.018852479250772179175665854700127965770661830902099609375;0.0676629390913203732083047725609503686428070068359375;0.0215218221916482869982001346897959592752158641815185546875;-0.3337719597552732953005261151702143251895904541015625;-0.557217789440134669831650171545334160327911376953125;0.58441348521352176614840345791890285909175872802734375;0.2448941135565920468319944802715326659381389617919921875;-0.2318118794441382524151862298822379671037197113037109375;-0.409878552480622049269953777184127829968929290771484375;-0.227154705872391726728665162227116525173187255859375]
      mask Display: e = 0.06;c1 = [120 150 230]/255;c2 = ([120 150 230]+0)/383;c3 = ([120 150 230]+128)/383;patch([0 1 1 0],[0 0 1 1],c1);patch([0 1 1 1-e 1-e 0+e],[0 0 1 1-e 0+e 0+e],c2);patch([0 0 1 1-e 0+e 0+e],[0 1 1 1-e 1-e 0+e],c3);plot([0 1 1 0 0],[0 0 1 1 0]);disp('weights');
  Mux : Mux  Inputs=20
      mask Display: e = 0.06;c1 = [120 150 230]/255;c2 = ([120 150 230]+0)/383;c3 = ([120 150 230]+128)/383;patch([0 1 1 0],[0 0 1 1],c1);patch([0 1 1 1-e 1-e 0+e],[0 0 1 1-e 0+e 0+e],c2);patch([0 0 1 1-e 0+e 0+e],[0 1 1 1-e 1-e 0+e],c3);plot([0 1 1 0 0],[0 0 1 1 0]);disp('Mux');
  dotprod{1..20} (20 alike) : neural/Weight Functions/dotprod
  "lz{2,1}" : Outport
      mask Display: e = 0.06;c1 = [120 230 180]/255;c2 = ([120 230 180]+0)/(256+128);c3 = ([120 230 180]+128)/(256+128);patch([0 1 1 0],[0 0 1 1],c1);patch([0 1 1 1-e 1-e 0+e],[0 0 1 1-e 0+e 0+e],c2);patch([0 0 1 1-e 0+e 0+e],[0 1 1 1-e 1-e 0+e],c3);plot([0 1 1 0 0],[0 0 1 1 0]);
  wires:
  "IW{2,1}(1,:)'"/1 -> dotprod1/1
  "ad{2,1}"/1 -> dotprod1/2, dotprod2/2, dotprod3/2, dotprod4/2, dotprod5/2, dotprod6/2, dotprod7/2, dotprod8/2, dotprod9/2, dotprod10/2, dotprod11/2, dotprod12/2, dotprod13/2, dotprod14/2, dotprod15/2, dotprod16/2, dotprod17/2, dotprod18/2, dotprod19/2, dotprod20/2
  dotprod1/1 -> Mux/1
  "IW{2,1}(2,:)'"/1 -> dotprod2/1
  dotprod2/1 -> Mux/2
  "IW{2,1}(3,:)'"/1 -> dotprod3/1
  dotprod3/1 -> Mux/3
  "IW{2,1}(4,:)'"/1 -> dotprod4/1
  dotprod4/1 -> Mux/4
  "IW{2,1}(5,:)'"/1 -> dotprod5/1
  dotprod5/1 -> Mux/5
  "IW{2,1}(6,:)'"/1 -> dotprod6/1
  dotprod6/1 -> Mux/6
  "IW{2,1}(7,:)'"/1 -> dotprod7/1
  dotprod7/1 -> Mux/7
  "IW{2,1}(8,:)'"/1 -> dotprod8/1
  dotprod8/1 -> Mux/8
  "IW{2,1}(9,:)'"/1 -> dotprod9/1
  dotprod9/1 -> Mux/9
  "IW{2,1}(10,:)'"/1 -> dotprod10/1
  dotprod10/1 -> Mux/10
  "IW{2,1}(11,:)'"/1 -> dotprod11/1
  dotprod11/1 -> Mux/11
  "IW{2,1}(12,:)'"/1 -> dotprod12/1
  dotprod12/1 -> Mux/12
  "IW{2,1}(13,:)'"/1 -> dotprod13/1
  dotprod13/1 -> Mux/13
  "IW{2,1}(14,:)'"/1 -> dotprod14/1
  dotprod14/1 -> Mux/14
  "IW{2,1}(15,:)'"/1 -> dotprod15/1
  dotprod15/1 -> Mux/15
  "IW{2,1}(16,:)'"/1 -> dotprod16/1
  dotprod16/1 -> Mux/16
  "IW{2,1}(17,:)'"/1 -> dotprod17/1
  dotprod17/1 -> Mux/17
  "IW{2,1}(18,:)'"/1 -> dotprod18/1
  dotprod18/1 -> Mux/18
  "IW{2,1}(19,:)'"/1 -> dotprod19/1
  dotprod19/1 -> Mux/19
  "IW{2,1}(20,:)'"/1 -> dotprod20/1
  dotprod20/1 -> Mux/20
  Mux/1 -> "lz{2,1}"/1

== system MCVT_Integration/Sys2 - Structural System/Sys2_Structural_System/Fault Detection and Diagnosis (FDD) 2.3/Pattern Recognition Neural Network/Pattern Recognition Neural Network with 10% Noise/Layer 3/Delays 1 ==
  "p{3}" : Inport  PortDimensions=20
      mask Display: e = 0.06;c1 = [120 230 180]/255;c2 = ([120 230 180]+0)/(256+128);c3 = ([120 230 180]+128)/(256+128);patch([0 1 1 0],[0 0 1 1],c1);patch([0 1 1 1-e 1-e 0+e],[0 0 1 1-e 0+e 0+e],c2);patch([0 0 1 1-e 0+e 0+e],[0 1 1 1-e 1-e 0+e],c3);plot([0 1 1 0 0],[0 0 1 1 0]);
  mux : Mux  Inputs=1
      mask Display: e = 0.06;c1 = [120 150 230]/255;c2 = ([120 150 230]+0)/383;c3 = ([120 150 230]+128)/383;patch([0 1 1 0],[0 0 1 1],c1);patch([0 1 1 1-e 1-e 0+e],[0 0 1 1-e 0+e 0+e],c2);patch([0 0 1 1-e 0+e 0+e],[0 1 1 1-e 1-e 0+e],c3);plot([0 1 1 0 0],[0 0 1 1 0]);disp('Mux')
  "pd{3,2}" : Outport
      mask Display: e = 0.06;c1 = [120 230 180]/255;c2 = ([120 230 180]+0)/(256+128);c3 = ([120 230 180]+128)/(256+128);patch([0 1 1 0],[0 0 1 1],c1);patch([0 1 1 1-e 1-e 0+e],[0 0 1 1-e 0+e 0+e],c2);patch([0 0 1 1-e 0+e 0+e],[0 1 1 1-e 1-e 0+e],c3);plot([0 1 1 0 0],[0 0 1 1 0]);
  wires:
  "p{3}"/1 -> mux/1
  mux/1 -> "pd{3,2}"/1

== system MCVT_Integration/Sys2 - Structural System/Sys2_Structural_System/Fault Detection and Diagnosis (FDD) 2.3/Pattern Recognition Neural Network/Pattern Recognition Neural Network with 10% Noise/Layer 3/LW{3,2} ==
  "ad{3,2}" : Inport  PortDimensions=20
      mask Display: e = 0.06;c1 = [120 230 180]/255;c2 = ([120 230 180]+0)/(256+128);c3 = ([120 230 180]+128)/(256+128);patch([0 1 1 0],[0 0 1 1],c1);patch([0 1 1 1-e 1-e 0+e],[0 0 1 1-e 0+e 0+e],c2);patch([0 0 1 1-e 0+e 0+e],[0 1 1 1-e 1-e 0+e],c3);plot([0 1 1 0 0],[0 0 1 1 0]);
  "IW{3,2}(1,:)'" : Constant  Value=[-0.46597240255782057527511597072589211165904998779296875;-0.613321676125609283047879216610454022884368896484375;-0.28437777983748435683963862175005488097667694091796875;1.4324045825755564731451840998488478362560272216796875;0.7970896775974380954465914328466169536113739013671875;1.3870791174102687914881926189991645514965057373046875;-0.97438388643140594336244930673274211585521697998046875;1.1079358057310908503012569781276397407054901123046875;-0.59566438295109713774166948496713303029537200927734375;-0.79514050824820203811071905874996446073055267333984375;0.325110412837921025630549820562009699642658233642578125;0.58678738807935049148767348015098832547664642333984375;-0.28678319750228287166038398936507292091846466064453125;0.5761307567965123777042890651500783860683441162109375;-0.30009424073517987352488489705137908458709716796875;0.3647370399169054255850141998962499201297760009765625;0.246495432388269486434495547655387781560420989990234375;0.8315821325752619230797790805809199810028076171875;-1.21059319111313623551495766150765120983123779296875;-0.5967411631203696220637766600702889263629913330078125]
      mask Display: e = 0.06;c1 = [120 150 230]/255;c2 = ([120 150 230]+0)/383;c3 = ([120 150 230]+128)/383;patch([0 1 1 0],[0 0 1 1],c1);patch([0 1 1 1-e 1-e 0+e],[0 0 1 1-e 0+e 0+e],c2);patch([0 0 1 1-e 0+e 0+e],[0 1 1 1-e 1-e 0+e],c3);plot([0 1 1 0 0],[0 0 1 1 0]);disp('weights');
  "IW{3,2}(2,:)'" : Constant  Value=[0.8708044347959236208822630942449904978275299072265625;-0.765646819559831381951653384021483361721038818359375;0.32036715500720924776345555073930881917476654052734375;0.777458126939139848587956294068135321140289306640625;0.477246839106822695608656204058206640183925628662109375;-0.497765996210863204129992709567886777222156524658203125;-0.38340962292213498496806778348400257527828216552734375;-1.0809441504940908718168657287606038153171539306640625;-0.6111228162078223835607104774680919945240020751953125;0.09705379665500617203388600273683550767600536346435546875;0.15651048232466013843122709658928215503692626953125;-0.49961124472076356983762934760306961834430694580078125;0.82979428869634930254761684409459121525287628173828125;-0.912753301536618710798620668356306850910186767578125;-1.5612420710330756623562820095685310661792755126953125;0.54667216246949601465843215919448994100093841552734375;-0.72291984141035381394857495251926593482494354248046875;0.65284231914790724626840301425545476377010345458984375;0.452509187551427383144897476086043752729892730712890625;-0.353049212127228562163594460798776708543300628662109375]
      mask Display: e = 0.06;c1 = [120 150 230]/255;c2 = ([120 150 230]+0)/383;c3 = ([120 150 230]+128)/383;patch([0 1 1 0],[0 0 1 1],c1);patch([0 1 1 1-e 1-e 0+e],[0 0 1 1-e 0+e 0+e],c2);patch([0 0 1 1-e 0+e 0+e],[0 1 1 1-e 1-e 0+e],c3);plot([0 1 1 0 0],[0 0 1 1 0]);disp('weights');
  "IW{3,2}(3,:)'" : Constant  Value=[-0.1345677352345765187546788865802227519452571868896484375;-0.323122071152291356899155516657629050314426422119140625;-0.010567255036895366326898937359146657399833202362060546875;-0.2392392912650132263951974209703621454536914825439453125;-1.5505013516066814549532182354596443474292755126953125;-0.13694663650523508646728032545070163905620574951171875;-0.5907848747451065474223241835716180503368377685546875;-0.65956618461376248507832542600226588547229766845703125;0.07430840079002225262438940944775822572410106658935546875;-1.0400862725712165524072361222351901233196258544921875;0.62883281845853955527303469352773390710353851318359375;0.252298084700958435977469207500689662992954254150390625;-0.50325594760295888629997307361918501555919647216796875;-1.1118294704349993562431109239696525037288665771484375;0.88850519292027296813785142148844897747039794921875;-0.95471726158567904452212360411067493259906768798828125;0.20982519989503012336484744082554243505001068115234375;-0.8373759218934420101021487425896339118480682373046875;-0.363473449556406313920575712472782470285892486572265625;-0.05268649560203829407267761553157470189034938812255859375]
      mask Display: e = 0.06;c1 = [120 150 230]/255;c2 = ([120 150 230]+0)/383;c3 = ([120 150 230]+128)/383;patch([0 1 1 0],[0 0 1 1],c1);patch([0 1 1 1-e 1-e 0+e],[0 0 1 1-e 0+e 0+e],c2);patch([0 0 1 1-e 0+e 0+e],[0 1 1 1-e 1-e 0+e],c3);plot([0 1 1 0 0],[0 0 1 1 0]);disp('weights');
  "IW{3,2}(4,:)'" : Constant  Value=[-1.182842004109295519498346038744784891605377197265625;-0.38282815577807038920354898436926305294036865234375;-1.010860191566054222533921347348950803279876708984375;-0.8788695169950171415251816142699681222438812255859375;-0.6798076731408022599367768634692765772342681884765625;0.4797653385151168237143792794086039066314697265625;-0.61573838214958309666968716555857099592685699462890625;-0.0013813284649994293958041868819464070838876068592071533203125;-0.301191662090578404775698118100990541279315948486328125;0.80978488616218091689091806983924470841884613037109375;-0.62406077947526072957629139636992476880550384521484375;-1.2790338645001873008055781610892154276371002197265625;0.7510018868821848325723067318904213607311248779296875;-0.2216541476419210121573399874250753782689571380615234375;0.0147236967101859635154159633430026588030159473419189453125;0.2200023488615381050959030062585952691733837127685546875;0.242153702472233167686255228545633144676685333251953125;-0.435298244511466536632582347010611556470394134521484375;1.132568543664171034635046453331597149372100830078125;1.1484415824454738963567024256917648017406463623046875]
      mask Display: e = 0.06;c1 = [120 150 230]/255;c2 = ([120 150 230]+0)/383;c3 = ([120 150 230]+128)/383;patch([0 1 1 0],[0 0 1 1],c1);patch([0 1 1 1-e 1-e 0+e],[0 0 1 1-e 0+e 0+e],c2);patch([0 0 1 1-e 0+e 0+e],[0 1 1 1-e 1-e 0+e],c3);plot([0 1 1 0 0],[0 0 1 1 0]);disp('weights');
  "IW{3,2}(5,:)'" : Constant  Value=[1.1754247525333314516871041632839478552341461181640625;0.57666970564611486071981971690547652542591094970703125;0.79416981787272089565732358096283860504627227783203125;-0.72459507178142812389154414631775580346584320068359375;0.65448248204684145168386066870880313217639923095703125;0.12473913750347530304818377544506802223622798919677734375;0.0868397224325205829842388993711210787296295166015625;0.4957524101809756000847073664772324264049530029296875;-0.38772243307517262334016550084925256669521331787109375;1.3273361050877767741695834047277458012104034423828125;-1.116214411653183358197338748141191899776458740234375;0.08578022349878493268970913732118788175284862518310546875;-0.937901738130868967147080184076912701129913330078125;0.511299389849998675572351203300058841705322265625;1.096342520648214691192379177664406597614288330078125;-0.4876935788915177649727183961658738553524017333984375;0.404888444888093612661350562120787799358367919921875;0.46089147636484906200848854496143758296966552734375;-0.9932317543524813441280230108532123267650604248046875;0.5851305970368338993381485124700702726840972900390625]
      mask Display: e = 0.06;c1 = [120 150 230]/255;c2 = ([120 150 230]+0)/383;c3 = ([120 150 230]+128)/383;patch([0 1 1 0],[0 0 1 1],c1);patch([0 1 1 1-e 1-e 0+e],[0 0 1 1-e 0+e 0+e],c2);patch([0 0 1 1-e 0+e 0+e],[0 1 1 1-e 1-e 0+e],c3);plot([0 1 1 0 0],[0 0 1 1 0]);disp('weights');
  "IW{3,2}(6,:)'" : Constant  Value=[-0.625181505761720046621121582575142383575439453125;0.74050495608783373224781598764820955693721771240234375;-1.2787719945095139539859019350842572748661041259765625;-0.265730819297855302973943025790504179894924163818359375;0.396127616349532141182265831957920454442501068115234375;0.45276775318712536577692162609309889376163482666015625;1.6991717021669103804271117041935212910175323486328125;0.39791416341121432775906896495143882930278778076171875;-0.9366432033540643686819748836569488048553466796875;-0.585010174871845922695001718238927423954010009765625;-0.89515293677175211684016176150180399417877197265625;-0.328317812309258771907849450144567526876926422119140625;0.63147804199407564507140477871871553361415863037109375;0.1908691686135129128576437551600974984467029571533203125;0.0944116327686489176240769438663846813142299652099609375;-0.11626155823480595341568033518342417664825916290283203125;-0.1344343205664212670402690719129168428480625152587890625;-1.0200680905062800007954137981869280338287353515625;0.3556248580565453121238306266604922711849212646484375;-0.87952593673225709647311987282591871917247772216796875]
      mask Display: e = 0.06;c1 = [120 150 230]/255;c2 = ([120 150 230]+0)/383;c3 = ([120 150 230]+128)/383;patch([0 1 1 0],[0 0 1 1],c1);patch([0 1 1 1-e 1-e 0+e],[0 0 1 1-e 0+e 0+e],c2);patch([0 0 1 1-e 0+e 0+e],[0 1 1 1-e 1-e 0+e],c3);plot([0 1 1 0 0],[0 0 1 1 0]);disp('weights');
  Mux : Mux  Inputs=6
      mask Display: e = 0.06;c1 = [120 150 230]/255;c2 = ([120 150 230]+0)/383;c3 = ([120 150 230]+128)/383;patch([0 1 1 0],[0 0 1 1],c1);patch([0 1 1 1-e 1-e 0+e],[0 0 1 1-e 0+e 0+e],c2);patch([0 0 1 1-e 0+e 0+e],[0 1 1 1-e 1-e 0+e],c3);plot([0 1 1 0 0],[0 0 1 1 0]);disp('Mux');
  dotprod{1..6} (6 alike) : neural/Weight Functions/dotprod
  "lz{3,2}" : Outport
      mask Display: e = 0.06;c1 = [120 230 180]/255;c2 = ([120 230 180]+0)/(256+128);c3 = ([120 230 180]+128)/(256+128);patch([0 1 1 0],[0 0 1 1],c1);patch([0 1 1 1-e 1-e 0+e],[0 0 1 1-e 0+e 0+e],c2);patch([0 0 1 1-e 0+e 0+e],[0 1 1 1-e 1-e 0+e],c3);plot([0 1 1 0 0],[0 0 1 1 0]);
  wires:
  "IW{3,2}(1,:)'"/1 -> dotprod1/1
  "ad{3,2}"/1 -> dotprod1/2, dotprod2/2, dotprod3/2, dotprod4/2, dotprod5/2, dotprod6/2
  dotprod1/1 -> Mux/1
  "IW{3,2}(2,:)'"/1 -> dotprod2/1
  dotprod2/1 -> Mux/2
  "IW{3,2}(3,:)'"/1 -> dotprod3/1
  dotprod3/1 -> Mux/3
  "IW{3,2}(4,:)'"/1 -> dotprod4/1
  dotprod4/1 -> Mux/4
  "IW{3,2}(5,:)'"/1 -> dotprod5/1
  dotprod5/1 -> Mux/5
  "IW{3,2}(6,:)'"/1 -> dotprod6/1
  dotprod6/1 -> Mux/6
  Mux/1 -> "lz{3,2}"/1

== system MCVT_Integration/Sys2 - Structural System/Sys2_Structural_System/Fault Detection and Diagnosis (FDD) with Binary Classifer/Accelerometer/Accelerometer/Switch ON//OFF Control Block/Switch OFF ==
  Sensor : Inport
  All Acc : Inport  Port=2
  Clock1 : Clock
  MATLAB Function1 : MATLAB Function  TreatAsAtomicUnit=on
  No Signal : Scope
  Selected Acc : Outport  VectorParamsAs1DForOutWhenUnconnected=off
  wires:
  Sensor/1 -> MATLAB Function1/1(sensor)
  MATLAB Function1/1(y) -> No Signal/1, Selected Acc/1
  Clock1/1 -> MATLAB Function1/3(Time)
  All Acc/1 -> MATLAB Function1/2(u)

== system MCVT_Integration/Sys2 - Structural System/Sys2_Structural_System/Fault Detection and Diagnosis (FDD) with Binary Classifer/Accelerometer/Accelerometer/Switch ON//OFF Control Block/Switch ON ==
  In1 : Inport
  Gain2 : Gain
  Signal Received : Scope
  ON : Outport  VectorParamsAs1DForOutWhenUnconnected=off
  wires:
  In1/1 -> Gain2/1
  Gain2/1 -> Signal Received/1, ON/1
